simulink model "BswSigProc"  (R2022b)
settings: Solver=FixedStepDiscrete  StartTime=0.0  StopTime=10.0  FixedStep=0.01  RelTol=1e-3
model workspace (MAT data in the model file):
  NvMBlockRecordSignal : Simulink.Signal (value not decoded)
  NvMBlockRecordSignalInit : Simulink.Parameter (value not decoded)
symbols that are objects in the model workspace (value not decoded): NvMBlockRecordSignalInit
(part 2 of 2)

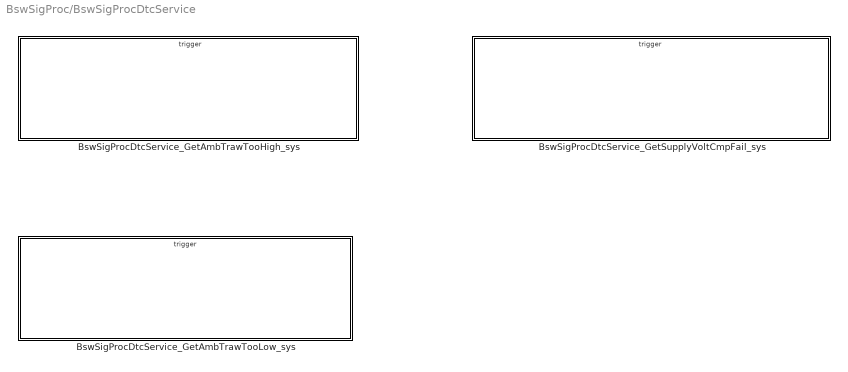
== system BswSigProc/BswSigProcDtcService ==
  BswSigProcDtcService_GetAmbTrawTooHigh_sys : Subsystem
  BswSigProcDtcService_GetAmbTrawTooLow_sys : Subsystem
  BswSigProcDtcService_GetSupplyVoltCmpFail_sys : Subsystem

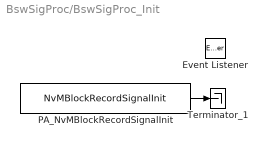
== system BswSigProc/BswSigProc_Init ==
  Event Listener : EventListener  EventName=reset
  PA_NvMBlockRecordSignalInit : Constant  Value=NvMBlockRecordSignalInit
  Terminator_1 : Terminator
  wires:
  PA_NvMBlockRecordSignalInit/1 -> Terminator_1/1

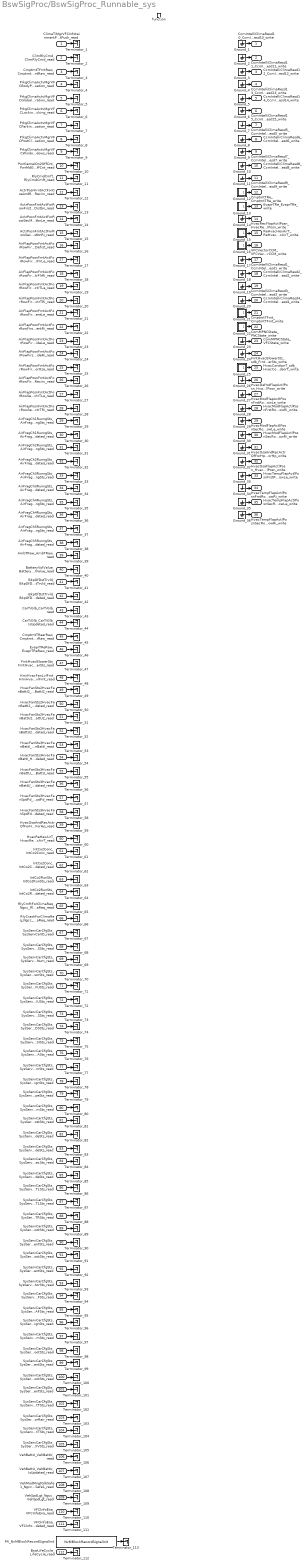
== system BswSigProc/BswSigProc_Runnable_sys ==
  ClimaTiMgrVFCInfotainmentPush_ClimaTiMgrVFCInfotainmentPush_read : Inport
  ClimRlyCmd_ClimRlyCmd_read : Inport  Port=2
  CmptmtTFrntRaw_CmptmtTFrntRaw_read : Inport  Port=3
  PrkgClimaActvMgrVFCBodyPreClimatisation_PrkgClimaActvMgrVFCBodyPreClimatisation_read : Inport  Port=4
  PrkgClimaActvMgrVFCGlobalShortDuration_PrkgClimaActvMgrVFCGlobalShortDuration_read : Inport  Port=5
  PrkgClimaActvMgrVFCLocking_PrkgClimaActvMgrVFCLocking_read : Inport  Port=6
  PrkgClimaActvMgrVFCParkingDrivingClimatization_PrkgClimaActvMgrVFCParkingDrivingClimatization_read : Inport  Port=7
  PrkgClimaActvMgrVFCPostClimatisation_PrkgClimaActvMgrVFCPostClimatisation_read : Inport  Port=8
  PrkgClimaActvMgrVFCWindows_PrkgClimaActvMgrVFCWindows_read : Inport  Port=9
  PwrGernalOn2OffCnt_PwrMdGernalOn2OffCnt_read : Inport  Port=10
  RlyCmdCmft_RlyCmdCmft_read : Inport  Port=11
  ActrPosnFrntActForOsaAndRecirc_ActrPosnFrntActForOsaAndRecirc_read : Inport  Port=12
  ActrPosnFrntActForRowFirstModDistbn_ActrPosnFrntActForRowFirstModDistbn_read : Inport  Port=13
  ActrPosnFrntActForRowSecModDistbnLe_ActrPosnFrntActForRowSecModDistbnLe_read : Inport  Port=14
  ActrPosnFrntActForRowSecModDistbnRi_ActrPosnFrntActForRowSecModDistbnRi_read : Inport  Port=15
  AirFlapPosnFrntActForRowFirstForActrDefrst_AirFlapPosnFrntActForRowFirstForActrDefrst_read : Inport  Port=16
  AirFlapPosnFrntActForRowFirstForActrFlrLe_AirFlapPosnFrntActForRowFirstForActrFlrLe_read : Inport  Port=17
  AirFlapPosnFrntActForRowFirstForActrFlrRi_AirFlapPosnFrntActForRowFirstForActrFlrRi_read : Inport  Port=18
  AirFlapPosnFrntActForRowFirstForActrTLe_AirFlapPosnFrntActForRowFirstForActrTLe_read : Inport  Port=19
  AirFlapPosnFrntActForRowFirstForActrTRi_AirFlapPosnFrntActForRowFirstForActrTRi_read : Inport  Port=20
  AirFlapPosnFrntActForRowFirstForActrVentLe_AirFlapPosnFrntActForRowFirstForActrVentLe_read : Inport  Port=21
  AirFlapPosnFrntActForRowFirstForActrVentRi_AirFlapPosnFrntActForRowFirstForActrVentRi_read : Inport  Port=22
  AirFlapPosnFrntActForRowFirstForActrVentSideLe_AirFlapPosnFrntActForRowFirstForActrVentSideLe_read : Inport  Port=23
  AirFlapPosnFrntActForRowFirstForActrVentSideRi_AirFlapPosnFrntActForRowFirstForActrVentSideRi_read : Inport  Port=24
  AirFlapPosnFrntActForRowFirstForOsa_AirFlapPosnFrntActForRowFirstForOsa_read : Inport  Port=25
  AirFlapPosnFrntActForRowFirstForRecirc_AirFlapPosnFrntActForRowFirstForRecirc_read : Inport  Port=26
  AirFlapPosnFrntActForRowSecForActrTLe_AirFlapPosnFrntActForRowSecForActrTLe_read : Inport  Port=27
  AirFlapPosnFrntActForRowSecForActrTRi_AirFlapPosnFrntActForRowSecForActrTRi_read : Inport  Port=28
  AirFragCh1RunngSts_AirFragCh1RunngSts_read : Inport  Port=29
  AirFragCh1RunngSts_AirFragCh1RunngSts_IsUpdated_read : Inport  Port=30
  AirFragCh2RunngSts_AirFragCh2RunngSts_read : Inport  Port=31
  AirFragCh2RunngSts_AirFragCh2RunngSts_IsUpdated_read : Inport  Port=32
  AirFragCh3RunngSts_AirFragCh3RunngSts_read : Inport  Port=33
  AirFragCh3RunngSts_AirFragCh3RunngSts_IsUpdated_read : Inport  Port=34
  AirFragCh4RunngSts_AirFragCh4RunngSts_read : Inport  Port=35
  AirFragCh4RunngSts_AirFragCh4RunngSts_IsUpdated_read : Inport  Port=36
  AirFragCh5RunngSts_AirFragCh5RunngSts_read : Inport  Port=37
  AirFragCh5RunngSts_AirFragCh5RunngSts_IsUpdated_read : Inport  Port=38
  AmbTRaw_AmbTRaw_read : Inport  Port=39
  BatteryVolValue_BatteryVolValue_read : Inport  Port=40
  BkpOfDstTrvld_BkpOfDstTrvld_read : Inport  Port=41
  BkpOfDstTrvld_BkpOfDstTrvld_IsUpdated_read : Inport  Port=42
  CarTiGlb_CarTiGlb_read : Inport  Port=43
  CarTiGlb_CarTiGlb_IsUpdated_read : Inport  Port=44
  CmptmtTRearRaw_CmptmtTRearRaw_read : Inport  Port=45
  EvaprTReRaw_EvaprTReRaw_read : Inport  Port=46
  FrntHvacBlowerSts_FrntHvacBlowerSts_read : Inport  Port=47
  HmiHvacFanLvlFrnt_HmiHvacFanLvlFrnt_read : Inport  Port=48
  HvacFanSts2HvacFanBattI2_HvacFanSts2HvacFanBattI2_read : Inport  Port=49
  HvacFanSts2HvacFanBattI2_HvacFanSts2HvacFanBattI2_IsUpdated_read : Inport  Port=50
  HvacFanSts2HvacFanBattU2_HvacFanSts2HvacFanBattU2_read : Inport  Port=51
  HvacFanSts2HvacFanBattU2_HvacFanSts2HvacFanBattU2_IsUpdated_read : Inport  Port=52
  HvacFanSts3HvacFanBattI_HvacFanSts3HvacFanBattI_read : Inport  Port=53
  HvacFanSts3HvacFanBattI_HvacFanSts3HvacFanBattI_IsUpdated_read : Inport  Port=54
  HvacFanSts3HvacFanBattU_HvacFanSts3HvacFanBattU_read : Inport  Port=55
  HvacFanSts3HvacFanBattU_HvacFanSts3HvacFanBattU_IsUpdated_read : Inport  Port=56
  HvacFanSts3HvacFanSpdFd_HvacFanSts3HvacFanSpdFd_read : Inport  Port=57
  HvacFanSts3HvacFanSpdFd_HvacFanSts3HvacFanSpdFd_IsUpdated_read : Inport  Port=58
  HvacOsaAndRecActrQfForHp_HvacOsaAndRecActrQfForHp_read : Inport  Port=59
  HvacReHexAirT_HvacReHexAirT_read : Inport  Port=60
  IntCo2Conc_IntCo2Conc_read : Inport  Port=61
  IntCo2Conc_IntCo2Conc_IsUpdated_read : Inport  Port=62
  IntCo2RunSts_IntCo2RunSts_read : Inport  Port=63
  IntCo2RunSts_IntCo2RunSts_IsUpdated_read : Inport  Port=64
  RlyCmftForClimaReq_Ngcc_RlyCmftForClimaReq_read : Inport  Port=65
  RlyCrashForClimaReq_Ngcc_RlyCrashForClimaReq_read : Inport  Port=66
  SysServCarCfgSts_SysServCarID_read : Inport  Port=67
  SysServCarCfgSts_SysServCCPAQSSts_read : Inport  Port=68
  SysServCarCfgSts_SysServCCPBGMNum_read : Inport  Port=69
  SysServCarCfgSts_SysServCCPHEXSensorSts_read : Inport  Port=70
  SysServCarCfgSts_SysServCCPLin2AFUSts_read : Inport  Port=71
  SysServCarCfgSts_SysServCCPLin2AIUSts_read : Inport  Port=72
  SysServCarCfgSts_SysServCCPLin2AQSSts_read : Inport  Port=73
  SysServCarCfgSts_SysServCCPLin2CDSSts_read : Inport  Port=74
  SysServCarCfgSts_SysServCCPLin2PMSISts_read : Inport  Port=75
  SysServCarCfgSts_SysServCCPLin3VGASts_read : Inport  Port=76
  SysServCarCfgSts_SysServDUAirVentSnrSts_read : Inport  Port=77
  SysServCarCfgSts_SysServDUBlowerPwmSignSts_read : Inport  Port=78
  SysServCarCfgSts_SysServDUBlowerPwmSignTypeSts_read : Inport  Port=79
  SysServCarCfgSts_SysServDUBlowerPwmSts_read : Inport  Port=80
  SysServCarCfgSts_SysServDUDCDefrostSts_read : Inport  Port=81
  SysServCarCfgSts_SysServDUDCFLMdeSts_read : Inport  Port=82
  SysServCarCfgSts_SysServDUDCModeSts_read : Inport  Port=83
  SysServCarCfgSts_SysServDUDCRecSts_read : Inport  Port=84
  SysServCarCfgSts_SysServDUDCSModeSts_read : Inport  Port=85
  SysServCarCfgSts_SysServDUDCSTLSts_read : Inport  Port=86
  SysServCarCfgSts_SysServDUDCTLSts_read : Inport  Port=87
  SysServCarCfgSts_SysServDUDCTRSts_read : Inport  Port=88
  SysServCarCfgSts_SysServDUFLFootSts_read : Inport  Port=89
  SysServCarCfgSts_SysServDUFLVentSts_read : Inport  Port=90
  SysServCarCfgSts_SysServDUFRFootSts_read : Inport  Port=91
  SysServCarCfgSts_SysServDUFRVentSts_read : Inport  Port=92
  SysServCarCfgSts_SysServDUIncarMotorSts_read : Inport  Port=93
  SysServCarCfgSts_SysServDULin1HBMFSts_read : Inport  Port=94
  SysServCarCfgSts_SysServDULin4HFAFSts_read : Inport  Port=95
  SysServCarCfgSts_SysServDUReBlowerPwmSignSts_read : Inport  Port=96
  SysServCarCfgSts_SysServDUReBlowerPwmSts_read : Inport  Port=97
  SysServCarCfgSts_SysServDUSFLFootSts_read : Inport  Port=98
  SysServCarCfgSts_SysServDUSFLVentSts_read : Inport  Port=99
  SysServCarCfgSts_SysServDUSFRFootSts_read : Inport  Port=100
  SysServCarCfgSts_SysServDUSFRVentSts_read : Inport  Port=101
  SysServCarCfgSts_SysServDUSnsrForFCmptmtTSts_read : Inport  Port=102
  SysServCarCfgSts_SysServDUSnsrForRair_read : Inport  Port=103
  SysServCarCfgSts_SysServDUSnsrForRCmptmtTSts_read : Inport  Port=104
  SysServCarCfgSts_SysServLowSideRETXVSts_read : Inport  Port=105
  VehBattU_VehBattU_read : Inport  Port=106
  VehBattU_VehBattU_IsUpdated_read : Inport  Port=107
  VehModMngtGlbSafe1_Ngcc_VehModMngtGlbSafe1_read : Inport  Port=108
  VehSpdLgt_Ngcc_VehSpdLgt_read : Inport  Port=109
  VFCInfoEna_VFCInfoEna_read : Inport  Port=110
  VFCInfoEna_VFCInfoEna_IsUpdated_read : Inport  Port=111
  BswLifeCycle_LifeCycle_read : Inport  Port=112
  function : TriggerPort  TriggerType=function-call  VariantControl=(inherit)  SampleTimeType=periodic  SampleTime=-1
  Ground_{1..11,14,17..20,23..24,26..36} (29 alike) : Ground
  Ground_12 : Subsystem
  Ground_13 : Subsystem
  Ground_15 : Subsystem
  Ground_16 : Subsystem
  Ground_21 : Subsystem
  Ground_22 : Subsystem
  Ground_25 : Subsystem
  PA_NvMBlockRecordSignalInit : Constant  Value=NvMBlockRecordSignalInit
  Terminator_{1..113} (113 alike) : Terminator
  CcmIntelliClimaResd10_CcmIntelliClimaResd10_write : Outport
      settings: EnsureOutportIsVirtual=on
  CcmIntelliClimaResd11_CcmIntelliClimaResd11_write : Outport  Port=2
      settings: EnsureOutportIsVirtual=on
  CcmIntelliClimaResd12_CcmIntelliClimaResd12_write : Outport  Port=3
      settings: EnsureOutportIsVirtual=on
  CcmIntelliClimaResd13_CcmIntelliClimaResd13_write : Outport  Port=4
      settings: EnsureOutportIsVirtual=on
  CcmIntelliClimaResd14_CcmIntelliClimaResd14_write : Outport  Port=5
      settings: EnsureOutportIsVirtual=on
  CcmIntelliClimaResd15_CcmIntelliClimaResd15_write : Outport  Port=6
      settings: EnsureOutportIsVirtual=on
  CcmIntelliClimaResd5_CcmIntelliClimaResd5_write : Outport  Port=7
      settings: EnsureOutportIsVirtual=on
  CcmIntelliClimaResd6_CcmIntelliClimaResd6_write : Outport  Port=8
      settings: EnsureOutportIsVirtual=on
  CcmIntelliClimaResd7_CcmIntelliClimaResd7_write : Outport  Port=9
      settings: EnsureOutportIsVirtual=on
  CcmIntelliClimaResd8_CcmIntelliClimaResd8_write : Outport  Port=10
      settings: EnsureOutportIsVirtual=on
  CcmIntelliClimaResd9_CcmIntelliClimaResd9_write : Outport  Port=11
      settings: EnsureOutportIsVirtual=on
  CmptmtTRe_CmptmtTRe_write : Outport  Port=12
      settings: EnsureOutportIsVirtual=on
  EvaprTRe_EvaprTRe_write : Outport  Port=13
      settings: EnsureOutportIsVirtual=on
  HvacRecFlapActlPosn_HvacRecFlapActlPosn_write : Outport  Port=14
      settings: EnsureOutportIsVirtual=on
  ReHvacHexAirT_ReHvacHexAirT_write : Outport  Port=15
      settings: EnsureOutportIsVirtual=on
  VFCVectorCCM_VFCVectorCCM_write : Outport  Port=16
      settings: EnsureOutportIsVirtual=on
  CcmIntelliClimaResd1_CcmIntelliClimaResd1_write : Outport  Port=17
      settings: EnsureOutportIsVirtual=on
  CcmIntelliClimaResd2_CcmIntelliClimaResd2_write : Outport  Port=18
      settings: EnsureOutportIsVirtual=on
  CcmIntelliClimaResd3_CcmIntelliClimaResd3_write : Outport  Port=19
      settings: EnsureOutportIsVirtual=on
  CcmIntelliClimaResd4_CcmIntelliClimaResd4_write : Outport  Port=20
      settings: EnsureOutportIsVirtual=on
  CmptmtTFrnt_CmptmtTFrnt_write : Outport  Port=21
      settings: EnsureOutportIsVirtual=on
  ComMPNCState_PNCState_write : Outport  Port=22
      settings: EnsureOutportIsVirtual=on
  ComMPNCState_VFCState_write : Outport  Port=23
      settings: EnsureOutportIsVirtual=on
  FrntHvacBlowerSts_sdb_FrntHvacBlowerSts_write : Outport  Port=24
      settings: EnsureOutportIsVirtual=on
  HvacCondsorT_sdb_HvacCondsorT_write : Outport  Port=25
      settings: EnsureOutportIsVirtual=on
  HvacDefrstFlapActlPosn_HvacDefrstFlapActlPosn_write : Outport  Port=26
      settings: EnsureOutportIsVirtual=on
  HvacModFlapActlPosnFrstRowLe_HvacModFlapActlPosnFrstRowLe_write : Outport  Port=27
      settings: EnsureOutportIsVirtual=on
  HvacModFlapActlPosnFrstRowRi_HvacModFlapActlPosnFrstRowRi_write : Outport  Port=28
      settings: EnsureOutportIsVirtual=on
  HvacModFlapActlPosnSecRowLe_HvacModFlapActlPosnSecRowLe_write : Outport  Port=29
      settings: EnsureOutportIsVirtual=on
  HvacModFlapActlPosnSecRowRi_HvacModFlapActlPosnSecRowRi_write : Outport  Port=30
      settings: EnsureOutportIsVirtual=on
  HvacOsaAndRecActrQfForHp_sdb_HvacOsaAndRecActrQfForHp_write : Outport  Port=31
      settings: EnsureOutportIsVirtual=on
  HvacOsaFlapActlPosn_HvacOsaFlapActlPosn_write : Outport  Port=32
      settings: EnsureOutportIsVirtual=on
  HvacTempFlapActlPosnFrstRowLe_HvacTempFlapActlPosnFrstRowLe_write : Outport  Port=33
      settings: EnsureOutportIsVirtual=on
  HvacTempFlapActlPosnFrstRowRi_HvacTempFlapActlPosnFrstRowRi_write : Outport  Port=34
      settings: EnsureOutportIsVirtual=on
  HvacTempFlapActlPosnSecRowLe_HvacTempFlapActlPosnSecRowLe_write : Outport  Port=35
      settings: EnsureOutportIsVirtual=on
  HvacTempFlapActlPosnSecRowRi_HvacTempFlapActlPosnSecRowRi_write : Outport  Port=36
      settings: EnsureOutportIsVirtual=on
  wires:
  ClimaTiMgrVFCInfotainmentPush_ClimaTiMgrVFCInfotainmentPush_read/1 -> Terminator_1/1
  ClimRlyCmd_ClimRlyCmd_read/1 -> Terminator_2/1
  CmptmtTFrntRaw_CmptmtTFrntRaw_read/1 -> Terminator_3/1
  PrkgClimaActvMgrVFCBodyPreClimatisation_PrkgClimaActvMgrVFCBodyPreClimatisation_read/1 -> Terminator_4/1
  PrkgClimaActvMgrVFCGlobalShortDuration_PrkgClimaActvMgrVFCGlobalShortDuration_read/1 -> Terminator_5/1
  PrkgClimaActvMgrVFCLocking_PrkgClimaActvMgrVFCLocking_read/1 -> Terminator_6/1
  PrkgClimaActvMgrVFCParkingDrivingClimatization_PrkgClimaActvMgrVFCParkingDrivingClimatization_read/1 -> Terminator_7/1
  PrkgClimaActvMgrVFCPostClimatisation_PrkgClimaActvMgrVFCPostClimatisation_read/1 -> Terminator_8/1
  PrkgClimaActvMgrVFCWindows_PrkgClimaActvMgrVFCWindows_read/1 -> Terminator_9/1
  PwrGernalOn2OffCnt_PwrMdGernalOn2OffCnt_read/1 -> Terminator_10/1
  RlyCmdCmft_RlyCmdCmft_read/1 -> Terminator_11/1
  ActrPosnFrntActForOsaAndRecirc_ActrPosnFrntActForOsaAndRecirc_read/1 -> Terminator_12/1
  ActrPosnFrntActForRowFirstModDistbn_ActrPosnFrntActForRowFirstModDistbn_read/1 -> Terminator_13/1
  ActrPosnFrntActForRowSecModDistbnLe_ActrPosnFrntActForRowSecModDistbnLe_read/1 -> Terminator_14/1
  ActrPosnFrntActForRowSecModDistbnRi_ActrPosnFrntActForRowSecModDistbnRi_read/1 -> Terminator_15/1
  AirFlapPosnFrntActForRowFirstForActrDefrst_AirFlapPosnFrntActForRowFirstForActrDefrst_read/1 -> Terminator_16/1
  AirFlapPosnFrntActForRowFirstForActrFlrLe_AirFlapPosnFrntActForRowFirstForActrFlrLe_read/1 -> Terminator_17/1
  AirFlapPosnFrntActForRowFirstForActrFlrRi_AirFlapPosnFrntActForRowFirstForActrFlrRi_read/1 -> Terminator_18/1
  AirFlapPosnFrntActForRowFirstForActrTLe_AirFlapPosnFrntActForRowFirstForActrTLe_read/1 -> Terminator_19/1
  AirFlapPosnFrntActForRowFirstForActrTRi_AirFlapPosnFrntActForRowFirstForActrTRi_read/1 -> Terminator_20/1
  AirFlapPosnFrntActForRowFirstForActrVentLe_AirFlapPosnFrntActForRowFirstForActrVentLe_read/1 -> Terminator_21/1
  AirFlapPosnFrntActForRowFirstForActrVentRi_AirFlapPosnFrntActForRowFirstForActrVentRi_read/1 -> Terminator_22/1
  AirFlapPosnFrntActForRowFirstForActrVentSideLe_AirFlapPosnFrntActForRowFirstForActrVentSideLe_read/1 -> Terminator_23/1
  AirFlapPosnFrntActForRowFirstForActrVentSideRi_AirFlapPosnFrntActForRowFirstForActrVentSideRi_read/1 -> Terminator_24/1
  AirFlapPosnFrntActForRowFirstForOsa_AirFlapPosnFrntActForRowFirstForOsa_read/1 -> Terminator_25/1
  AirFlapPosnFrntActForRowFirstForRecirc_AirFlapPosnFrntActForRowFirstForRecirc_read/1 -> Terminator_26/1
  AirFlapPosnFrntActForRowSecForActrTLe_AirFlapPosnFrntActForRowSecForActrTLe_read/1 -> Terminator_27/1
  AirFlapPosnFrntActForRowSecForActrTRi_AirFlapPosnFrntActForRowSecForActrTRi_read/1 -> Terminator_28/1
  AirFragCh1RunngSts_AirFragCh1RunngSts_read/1 -> Terminator_29/1
  AirFragCh1RunngSts_AirFragCh1RunngSts_IsUpdated_read/1 -> Terminator_30/1
  AirFragCh2RunngSts_AirFragCh2RunngSts_read/1 -> Terminator_31/1
  AirFragCh2RunngSts_AirFragCh2RunngSts_IsUpdated_read/1 -> Terminator_32/1
  AirFragCh3RunngSts_AirFragCh3RunngSts_read/1 -> Terminator_33/1
  AirFragCh3RunngSts_AirFragCh3RunngSts_IsUpdated_read/1 -> Terminator_34/1
  AirFragCh4RunngSts_AirFragCh4RunngSts_read/1 -> Terminator_35/1
  AirFragCh4RunngSts_AirFragCh4RunngSts_IsUpdated_read/1 -> Terminator_36/1
  AirFragCh5RunngSts_AirFragCh5RunngSts_read/1 -> Terminator_37/1
  AirFragCh5RunngSts_AirFragCh5RunngSts_IsUpdated_read/1 -> Terminator_38/1
  AmbTRaw_AmbTRaw_read/1 -> Terminator_39/1
  BatteryVolValue_BatteryVolValue_read/1 -> Terminator_40/1
  BkpOfDstTrvld_BkpOfDstTrvld_read/1 -> Terminator_41/1
  BkpOfDstTrvld_BkpOfDstTrvld_IsUpdated_read/1 -> Terminator_42/1
  CarTiGlb_CarTiGlb_read/1 -> Terminator_43/1
  CarTiGlb_CarTiGlb_IsUpdated_read/1 -> Terminator_44/1
  CmptmtTRearRaw_CmptmtTRearRaw_read/1 -> Terminator_45/1
  EvaprTReRaw_EvaprTReRaw_read/1 -> Terminator_46/1
  FrntHvacBlowerSts_FrntHvacBlowerSts_read/1 -> Terminator_47/1
  HmiHvacFanLvlFrnt_HmiHvacFanLvlFrnt_read/1 -> Terminator_48/1
  HvacFanSts2HvacFanBattI2_HvacFanSts2HvacFanBattI2_read/1 -> Terminator_49/1
  HvacFanSts2HvacFanBattI2_HvacFanSts2HvacFanBattI2_IsUpdated_read/1 -> Terminator_50/1
  HvacFanSts2HvacFanBattU2_HvacFanSts2HvacFanBattU2_read/1 -> Terminator_51/1
  HvacFanSts2HvacFanBattU2_HvacFanSts2HvacFanBattU2_IsUpdated_read/1 -> Terminator_52/1
  HvacFanSts3HvacFanBattI_HvacFanSts3HvacFanBattI_read/1 -> Terminator_53/1
  HvacFanSts3HvacFanBattI_HvacFanSts3HvacFanBattI_IsUpdated_read/1 -> Terminator_54/1
  HvacFanSts3HvacFanBattU_HvacFanSts3HvacFanBattU_read/1 -> Terminator_55/1
  HvacFanSts3HvacFanBattU_HvacFanSts3HvacFanBattU_IsUpdated_read/1 -> Terminator_56/1
  HvacFanSts3HvacFanSpdFd_HvacFanSts3HvacFanSpdFd_read/1 -> Terminator_57/1
  HvacFanSts3HvacFanSpdFd_HvacFanSts3HvacFanSpdFd_IsUpdated_read/1 -> Terminator_58/1
  HvacOsaAndRecActrQfForHp_HvacOsaAndRecActrQfForHp_read/1 -> Terminator_59/1
  HvacReHexAirT_HvacReHexAirT_read/1 -> Terminator_60/1
  IntCo2Conc_IntCo2Conc_read/1 -> Terminator_61/1
  IntCo2Conc_IntCo2Conc_IsUpdated_read/1 -> Terminator_62/1
  IntCo2RunSts_IntCo2RunSts_read/1 -> Terminator_63/1
  IntCo2RunSts_IntCo2RunSts_IsUpdated_read/1 -> Terminator_64/1
  RlyCmftForClimaReq_Ngcc_RlyCmftForClimaReq_read/1 -> Terminator_65/1
  RlyCrashForClimaReq_Ngcc_RlyCrashForClimaReq_read/1 -> Terminator_66/1
  SysServCarCfgSts_SysServCarID_read/1 -> Terminator_67/1
  SysServCarCfgSts_SysServCCPAQSSts_read/1 -> Terminator_68/1
  SysServCarCfgSts_SysServCCPBGMNum_read/1 -> Terminator_69/1
  SysServCarCfgSts_SysServCCPHEXSensorSts_read/1 -> Terminator_70/1
  SysServCarCfgSts_SysServCCPLin2AFUSts_read/1 -> Terminator_71/1
  SysServCarCfgSts_SysServCCPLin2AIUSts_read/1 -> Terminator_72/1
  SysServCarCfgSts_SysServCCPLin2AQSSts_read/1 -> Terminator_73/1
  SysServCarCfgSts_SysServCCPLin2CDSSts_read/1 -> Terminator_74/1
  SysServCarCfgSts_SysServCCPLin2PMSISts_read/1 -> Terminator_75/1
  SysServCarCfgSts_SysServCCPLin3VGASts_read/1 -> Terminator_76/1
  SysServCarCfgSts_SysServDUAirVentSnrSts_read/1 -> Terminator_77/1
  SysServCarCfgSts_SysServDUBlowerPwmSignSts_read/1 -> Terminator_78/1
  SysServCarCfgSts_SysServDUBlowerPwmSignTypeSts_read/1 -> Terminator_79/1
  SysServCarCfgSts_SysServDUBlowerPwmSts_read/1 -> Terminator_80/1
  SysServCarCfgSts_SysServDUDCDefrostSts_read/1 -> Terminator_81/1
  SysServCarCfgSts_SysServDUDCFLMdeSts_read/1 -> Terminator_82/1
  SysServCarCfgSts_SysServDUDCModeSts_read/1 -> Terminator_83/1
  SysServCarCfgSts_SysServDUDCRecSts_read/1 -> Terminator_84/1
  SysServCarCfgSts_SysServDUDCSModeSts_read/1 -> Terminator_85/1
  SysServCarCfgSts_SysServDUDCSTLSts_read/1 -> Terminator_86/1
  SysServCarCfgSts_SysServDUDCTLSts_read/1 -> Terminator_87/1
  SysServCarCfgSts_SysServDUDCTRSts_read/1 -> Terminator_88/1
  SysServCarCfgSts_SysServDUFLFootSts_read/1 -> Terminator_89/1
  SysServCarCfgSts_SysServDUFLVentSts_read/1 -> Terminator_90/1
  SysServCarCfgSts_SysServDUFRFootSts_read/1 -> Terminator_91/1
  SysServCarCfgSts_SysServDUFRVentSts_read/1 -> Terminator_92/1
  SysServCarCfgSts_SysServDUIncarMotorSts_read/1 -> Terminator_93/1
  SysServCarCfgSts_SysServDULin1HBMFSts_read/1 -> Terminator_94/1
  SysServCarCfgSts_SysServDULin4HFAFSts_read/1 -> Terminator_95/1
  SysServCarCfgSts_SysServDUReBlowerPwmSignSts_read/1 -> Terminator_96/1
  SysServCarCfgSts_SysServDUReBlowerPwmSts_read/1 -> Terminator_97/1
  SysServCarCfgSts_SysServDUSFLFootSts_read/1 -> Terminator_98/1
  SysServCarCfgSts_SysServDUSFLVentSts_read/1 -> Terminator_99/1
  SysServCarCfgSts_SysServDUSFRFootSts_read/1 -> Terminator_100/1
  SysServCarCfgSts_SysServDUSFRVentSts_read/1 -> Terminator_101/1
  SysServCarCfgSts_SysServDUSnsrForFCmptmtTSts_read/1 -> Terminator_102/1
  SysServCarCfgSts_SysServDUSnsrForRair_read/1 -> Terminator_103/1
  SysServCarCfgSts_SysServDUSnsrForRCmptmtTSts_read/1 -> Terminator_104/1
  SysServCarCfgSts_SysServLowSideRETXVSts_read/1 -> Terminator_105/1
  VehBattU_VehBattU_read/1 -> Terminator_106/1
  VehBattU_VehBattU_IsUpdated_read/1 -> Terminator_107/1
  VehModMngtGlbSafe1_Ngcc_VehModMngtGlbSafe1_read/1 -> Terminator_108/1
  VehSpdLgt_Ngcc_VehSpdLgt_read/1 -> Terminator_109/1
  VFCInfoEna_VFCInfoEna_read/1 -> Terminator_110/1
  VFCInfoEna_VFCInfoEna_IsUpdated_read/1 -> Terminator_111/1
  BswLifeCycle_LifeCycle_read/1 -> Terminator_112/1
  PA_NvMBlockRecordSignalInit/1 -> Terminator_113/1
  Ground_1/1 -> CcmIntelliClimaResd10_CcmIntelliClimaResd10_write/1
  Ground_2/1 -> CcmIntelliClimaResd11_CcmIntelliClimaResd11_write/1
  Ground_3/1 -> CcmIntelliClimaResd12_CcmIntelliClimaResd12_write/1
  Ground_4/1 -> CcmIntelliClimaResd13_CcmIntelliClimaResd13_write/1
  Ground_5/1 -> CcmIntelliClimaResd14_CcmIntelliClimaResd14_write/1
  Ground_6/1 -> CcmIntelliClimaResd15_CcmIntelliClimaResd15_write/1
  Ground_7/1 -> CcmIntelliClimaResd5_CcmIntelliClimaResd5_write/1
  Ground_8/1 -> CcmIntelliClimaResd6_CcmIntelliClimaResd6_write/1
  Ground_9/1 -> CcmIntelliClimaResd7_CcmIntelliClimaResd7_write/1
  Ground_10/1 -> CcmIntelliClimaResd8_CcmIntelliClimaResd8_write/1
  Ground_11/1 -> CcmIntelliClimaResd9_CcmIntelliClimaResd9_write/1
  Ground_12/1(Out1) -> CmptmtTRe_CmptmtTRe_write/1
  Ground_13/1(Out1) -> EvaprTRe_EvaprTRe_write/1
  Ground_14/1 -> HvacRecFlapActlPosn_HvacRecFlapActlPosn_write/1
  Ground_15/1(Out1) -> ReHvacHexAirT_ReHvacHexAirT_write/1
  Ground_16/1(Out1) -> VFCVectorCCM_VFCVectorCCM_write/1
  Ground_17/1 -> CcmIntelliClimaResd1_CcmIntelliClimaResd1_write/1
  Ground_18/1 -> CcmIntelliClimaResd2_CcmIntelliClimaResd2_write/1
  Ground_19/1 -> CcmIntelliClimaResd3_CcmIntelliClimaResd3_write/1
  Ground_20/1 -> CcmIntelliClimaResd4_CcmIntelliClimaResd4_write/1
  Ground_21/1(Out1) -> CmptmtTFrnt_CmptmtTFrnt_write/1
  Ground_22/1(Out1) -> ComMPNCState_PNCState_write/1
  Ground_23/1 -> ComMPNCState_VFCState_write/1
  Ground_24/1 -> FrntHvacBlowerSts_sdb_FrntHvacBlowerSts_write/1
  Ground_25/1(Out1) -> HvacCondsorT_sdb_HvacCondsorT_write/1
  Ground_26/1 -> HvacDefrstFlapActlPosn_HvacDefrstFlapActlPosn_write/1
  Ground_27/1 -> HvacModFlapActlPosnFrstRowLe_HvacModFlapActlPosnFrstRowLe_write/1
  Ground_28/1 -> HvacModFlapActlPosnFrstRowRi_HvacModFlapActlPosnFrstRowRi_write/1
  Ground_29/1 -> HvacModFlapActlPosnSecRowLe_HvacModFlapActlPosnSecRowLe_write/1
  Ground_30/1 -> HvacModFlapActlPosnSecRowRi_HvacModFlapActlPosnSecRowRi_write/1
  Ground_31/1 -> HvacOsaAndRecActrQfForHp_sdb_HvacOsaAndRecActrQfForHp_write/1
  Ground_32/1 -> HvacOsaFlapActlPosn_HvacOsaFlapActlPosn_write/1
  Ground_33/1 -> HvacTempFlapActlPosnFrstRowLe_HvacTempFlapActlPosnFrstRowLe_write/1
  Ground_34/1 -> HvacTempFlapActlPosnFrstRowRi_HvacTempFlapActlPosnFrstRowRi_write/1
  Ground_35/1 -> HvacTempFlapActlPosnSecRowLe_HvacTempFlapActlPosnSecRowLe_write/1
  Ground_36/1 -> HvacTempFlapActlPosnSecRowRi_HvacTempFlapActlPosnSecRowRi_write/1

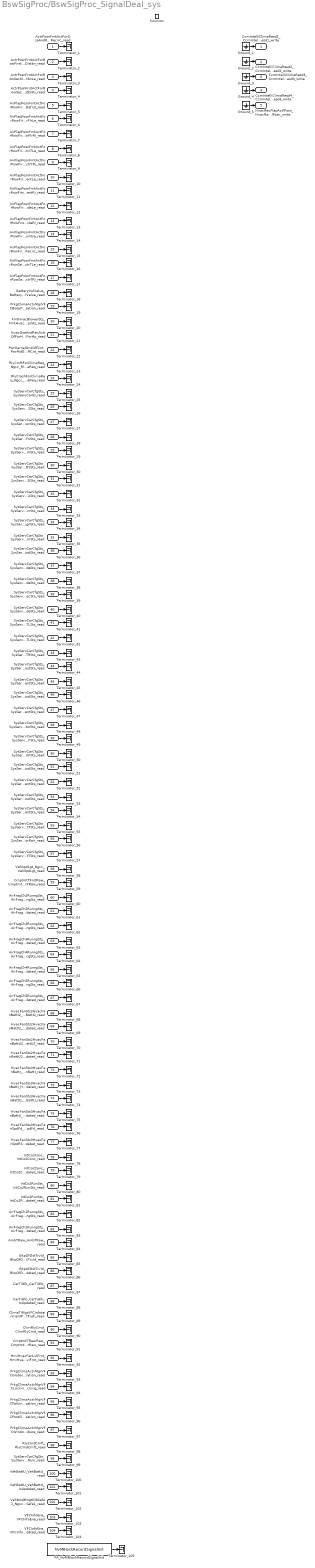
== system BswSigProc/BswSigProc_SignalDeal_sys ==
  ActrPosnFrntActForOsaAndRecirc_ActrPosnFrntActForOsaAndRecirc_read : Inport
  ActrPosnFrntActForRowFirstModDistbn_ActrPosnFrntActForRowFirstModDistbn_read : Inport  Port=2
  ActrPosnFrntActForRowSecModDistbnLe_ActrPosnFrntActForRowSecModDistbnLe_read : Inport  Port=3
  ActrPosnFrntActForRowSecModDistbnRi_ActrPosnFrntActForRowSecModDistbnRi_read : Inport  Port=4
  AirFlapPosnFrntActForRowFirstForActrDefrst_AirFlapPosnFrntActForRowFirstForActrDefrst_read : Inport  Port=5
  AirFlapPosnFrntActForRowFirstForActrFlrLe_AirFlapPosnFrntActForRowFirstForActrFlrLe_read : Inport  Port=6
  AirFlapPosnFrntActForRowFirstForActrFlrRi_AirFlapPosnFrntActForRowFirstForActrFlrRi_read : Inport  Port=7
  AirFlapPosnFrntActForRowFirstForActrTLe_AirFlapPosnFrntActForRowFirstForActrTLe_read : Inport  Port=8
  AirFlapPosnFrntActForRowFirstForActrTRi_AirFlapPosnFrntActForRowFirstForActrTRi_read : Inport  Port=9
  AirFlapPosnFrntActForRowFirstForActrVentLe_AirFlapPosnFrntActForRowFirstForActrVentLe_read : Inport  Port=10
  AirFlapPosnFrntActForRowFirstForActrVentRi_AirFlapPosnFrntActForRowFirstForActrVentRi_read : Inport  Port=11
  AirFlapPosnFrntActForRowFirstForActrVentSideLe_AirFlapPosnFrntActForRowFirstForActrVentSideLe_read : Inport  Port=12
  AirFlapPosnFrntActForRowFirstForActrVentSideRi_AirFlapPosnFrntActForRowFirstForActrVentSideRi_read : Inport  Port=13
  AirFlapPosnFrntActForRowFirstForOsa_AirFlapPosnFrntActForRowFirstForOsa_read : Inport  Port=14
  AirFlapPosnFrntActForRowFirstForRecirc_AirFlapPosnFrntActForRowFirstForRecirc_read : Inport  Port=15
  AirFlapPosnFrntActForRowSecForActrTLe_AirFlapPosnFrntActForRowSecForActrTLe_read : Inport  Port=16
  AirFlapPosnFrntActForRowSecForActrTRi_AirFlapPosnFrntActForRowSecForActrTRi_read : Inport  Port=17
  BatteryVolValue_BatteryVolValue_read : Inport  Port=18
  PrkgClimaActvMgrVFCBodyPreClimatisation_PrkgClimaActvMgrVFCBodyPreClimatisation_read : Inport  Port=19
  FrntHvacBlowerSts_FrntHvacBlowerSts_read : Inport  Port=20
  HvacOsaAndRecActrQfForHp_HvacOsaAndRecActrQfForHp_read : Inport  Port=21
  PwrGernalOn2OffCnt_PwrMdGernalOn2OffCnt_read : Inport  Port=22
  RlyCmftForClimaReq_Ngcc_RlyCmftForClimaReq_read : Inport  Port=23
  RlyCrashForClimaReq_Ngcc_RlyCrashForClimaReq_read : Inport  Port=24
  SysServCarCfgSts_SysServCarID_read : Inport  Port=25
  SysServCarCfgSts_SysServCCPAQSSts_read : Inport  Port=26
  SysServCarCfgSts_SysServCCPHEXSensorSts_read : Inport  Port=27
  SysServCarCfgSts_SysServCCPLin2AFUSts_read : Inport  Port=28
  SysServCarCfgSts_SysServCCPLin2AIUSts_read : Inport  Port=29
  SysServCarCfgSts_SysServCCPLin2CDSSts_read : Inport  Port=30
  SysServCarCfgSts_SysServCCPLin2PMSISts_read : Inport  Port=31
  SysServCarCfgSts_SysServCCPLin3VGASts_read : Inport  Port=32
  SysServCarCfgSts_SysServDUAirVentSnrSts_read : Inport  Port=33
  SysServCarCfgSts_SysServDUBlowerPwmSignSts_read : Inport  Port=34
  SysServCarCfgSts_SysServDUBlowerPwmSts_read : Inport  Port=35
  SysServCarCfgSts_SysServDUDCDefrostSts_read : Inport  Port=36
  SysServCarCfgSts_SysServDUDCFLMdeSts_read : Inport  Port=37
  SysServCarCfgSts_SysServDUDCModeSts_read : Inport  Port=38
  SysServCarCfgSts_SysServDUDCRecSts_read : Inport  Port=39
  SysServCarCfgSts_SysServDUDCSModeSts_read : Inport  Port=40
  SysServCarCfgSts_SysServDUDCSTLSts_read : Inport  Port=41
  SysServCarCfgSts_SysServDUDCTLSts_read : Inport  Port=42
  SysServCarCfgSts_SysServDUDCTRSts_read : Inport  Port=43
  SysServCarCfgSts_SysServDUFLFootSts_read : Inport  Port=44
  SysServCarCfgSts_SysServDUFLVentSts_read : Inport  Port=45
  SysServCarCfgSts_SysServDUFRFootSts_read : Inport  Port=46
  SysServCarCfgSts_SysServDUFRVentSts_read : Inport  Port=47
  SysServCarCfgSts_SysServDUIncarMotorSts_read : Inport  Port=48
  SysServCarCfgSts_SysServDULin1HBMFSts_read : Inport  Port=49
  SysServCarCfgSts_SysServDULin4HFAFSts_read : Inport  Port=50
  SysServCarCfgSts_SysServDUSFLFootSts_read : Inport  Port=51
  SysServCarCfgSts_SysServDUSFLVentSts_read : Inport  Port=52
  SysServCarCfgSts_SysServDUSFRFootSts_read : Inport  Port=53
  SysServCarCfgSts_SysServDUSFRVentSts_read : Inport  Port=54
  SysServCarCfgSts_SysServDUSnsrForFCmptmtTSts_read : Inport  Port=55
  SysServCarCfgSts_SysServDUSnsrForRair_read : Inport  Port=56
  SysServCarCfgSts_SysServDUSnsrForRCmptmtTSts_read : Inport  Port=57
  VehSpdLgt_Ngcc_VehSpdLgt_read : Inport  Port=58
  CmptmtTFrntRaw_CmptmtTFrntRaw_read : Inport  Port=59
  AirFragCh2RunngSts_AirFragCh2RunngSts_read : Inport  Port=60
  AirFragCh2RunngSts_AirFragCh2RunngSts_IsUpdated_read : Inport  Port=61
  AirFragCh3RunngSts_AirFragCh3RunngSts_read : Inport  Port=62
  AirFragCh3RunngSts_AirFragCh3RunngSts_IsUpdated_read : Inport  Port=63
  AirFragCh4RunngSts_AirFragCh4RunngSts_read : Inport  Port=64
  AirFragCh4RunngSts_AirFragCh4RunngSts_IsUpdated_read : Inport  Port=65
  AirFragCh5RunngSts_AirFragCh5RunngSts_read : Inport  Port=66
  AirFragCh5RunngSts_AirFragCh5RunngSts_IsUpdated_read : Inport  Port=67
  HvacFanSts2HvacFanBattI2_HvacFanSts2HvacFanBattI2_read : Inport  Port=68
  HvacFanSts2HvacFanBattI2_HvacFanSts2HvacFanBattI2_IsUpdated_read : Inport  Port=69
  HvacFanSts2HvacFanBattU2_HvacFanSts2HvacFanBattU2_read : Inport  Port=70
  HvacFanSts2HvacFanBattU2_HvacFanSts2HvacFanBattU2_IsUpdated_read : Inport  Port=71
  HvacFanSts3HvacFanBattI_HvacFanSts3HvacFanBattI_read : Inport  Port=72
  HvacFanSts3HvacFanBattI_HvacFanSts3HvacFanBattI_IsUpdated_read : Inport  Port=73
  HvacFanSts3HvacFanBattU_HvacFanSts3HvacFanBattU_read : Inport  Port=74
  HvacFanSts3HvacFanBattU_HvacFanSts3HvacFanBattU_IsUpdated_read : Inport  Port=75
  HvacFanSts3HvacFanSpdFd_HvacFanSts3HvacFanSpdFd_read : Inport  Port=76
  HvacFanSts3HvacFanSpdFd_HvacFanSts3HvacFanSpdFd_IsUpdated_read : Inport  Port=77
  IntCo2Conc_IntCo2Conc_read : Inport  Port=78
  IntCo2Conc_IntCo2Conc_IsUpdated_read : Inport  Port=79
  IntCo2RunSts_IntCo2RunSts_read : Inport  Port=80
  IntCo2RunSts_IntCo2RunSts_IsUpdated_read : Inport  Port=81
  AirFragCh1RunngSts_AirFragCh1RunngSts_read : Inport  Port=82
  AirFragCh1RunngSts_AirFragCh1RunngSts_IsUpdated_read : Inport  Port=83
  AmbTRaw_AmbTRaw_read : Inport  Port=84
  BkpOfDstTrvld_BkpOfDstTrvld_read : Inport  Port=85
  BkpOfDstTrvld_BkpOfDstTrvld_IsUpdated_read : Inport  Port=86
  CarTiGlb_CarTiGlb_read : Inport  Port=87
  CarTiGlb_CarTiGlb_IsUpdated_read : Inport  Port=88
  ClimaTiMgrVFCInfotainmentPush_ClimaTiMgrVFCInfotainmentPush_read : Inport  Port=89
  ClimRlyCmd_ClimRlyCmd_read : Inport  Port=90
  CmptmtTRearRaw_CmptmtTRearRaw_read : Inport  Port=91
  HmiHvacFanLvlFrnt_HmiHvacFanLvlFrnt_read : Inport  Port=92
  PrkgClimaActvMgrVFCGlobalShortDuration_PrkgClimaActvMgrVFCGlobalShortDuration_read : Inport  Port=93
  PrkgClimaActvMgrVFCLocking_PrkgClimaActvMgrVFCLocking_read : Inport  Port=94
  PrkgClimaActvMgrVFCParkingDrivingClimatization_PrkgClimaActvMgrVFCParkingDrivingClimatization_read : Inport  Port=95
  PrkgClimaActvMgrVFCPostClimatisation_PrkgClimaActvMgrVFCPostClimatisation_read : Inport  Port=96
  PrkgClimaActvMgrVFCWindows_PrkgClimaActvMgrVFCWindows_read : Inport  Port=97
  RlyCmdCmft_RlyCmdCmft_read : Inport  Port=98
  SysServCarCfgSts_SysServCCPBGMNum_read : Inport  Port=99
  VehBattU_VehBattU_read : Inport  Port=100
  VehBattU_VehBattU_IsUpdated_read : Inport  Port=101
  VehModMngtGlbSafe1_Ngcc_VehModMngtGlbSafe1_read : Inport  Port=102
  VFCInfoEna_VFCInfoEna_read : Inport  Port=103
  VFCInfoEna_VFCInfoEna_IsUpdated_read : Inport  Port=104
  function : TriggerPort  TriggerType=function-call  VariantControl=(inherit)  SampleTimeType=periodic  SampleTime=-1
  Ground_{1..5} (5 alike) : Ground
  PA_NvMBlockRecordSignalInit : Constant  Value=NvMBlockRecordSignalInit
  Terminator_{1..105} (105 alike) : Terminator
  CcmIntelliClimaResd1_CcmIntelliClimaResd1_write : Outport
      settings: EnsureOutportIsVirtual=on
  CcmIntelliClimaResd2_CcmIntelliClimaResd2_write : Outport  Port=2
      settings: EnsureOutportIsVirtual=on
  CcmIntelliClimaResd3_CcmIntelliClimaResd3_write : Outport  Port=3
      settings: EnsureOutportIsVirtual=on
  CcmIntelliClimaResd4_CcmIntelliClimaResd4_write : Outport  Port=4
      settings: EnsureOutportIsVirtual=on
  HvacRecFlapActlPosn_HvacRecFlapActlPosn_write : Outport  Port=5
      settings: EnsureOutportIsVirtual=on
  wires:
  ActrPosnFrntActForOsaAndRecirc_ActrPosnFrntActForOsaAndRecirc_read/1 -> Terminator_1/1
  ActrPosnFrntActForRowFirstModDistbn_ActrPosnFrntActForRowFirstModDistbn_read/1 -> Terminator_2/1
  ActrPosnFrntActForRowSecModDistbnLe_ActrPosnFrntActForRowSecModDistbnLe_read/1 -> Terminator_3/1
  ActrPosnFrntActForRowSecModDistbnRi_ActrPosnFrntActForRowSecModDistbnRi_read/1 -> Terminator_4/1
  AirFlapPosnFrntActForRowFirstForActrDefrst_AirFlapPosnFrntActForRowFirstForActrDefrst_read/1 -> Terminator_5/1
  AirFlapPosnFrntActForRowFirstForActrFlrLe_AirFlapPosnFrntActForRowFirstForActrFlrLe_read/1 -> Terminator_6/1
  AirFlapPosnFrntActForRowFirstForActrFlrRi_AirFlapPosnFrntActForRowFirstForActrFlrRi_read/1 -> Terminator_7/1
  AirFlapPosnFrntActForRowFirstForActrTLe_AirFlapPosnFrntActForRowFirstForActrTLe_read/1 -> Terminator_8/1
  AirFlapPosnFrntActForRowFirstForActrTRi_AirFlapPosnFrntActForRowFirstForActrTRi_read/1 -> Terminator_9/1
  AirFlapPosnFrntActForRowFirstForActrVentLe_AirFlapPosnFrntActForRowFirstForActrVentLe_read/1 -> Terminator_10/1
  AirFlapPosnFrntActForRowFirstForActrVentRi_AirFlapPosnFrntActForRowFirstForActrVentRi_read/1 -> Terminator_11/1
  AirFlapPosnFrntActForRowFirstForActrVentSideLe_AirFlapPosnFrntActForRowFirstForActrVentSideLe_read/1 -> Terminator_12/1
  AirFlapPosnFrntActForRowFirstForActrVentSideRi_AirFlapPosnFrntActForRowFirstForActrVentSideRi_read/1 -> Terminator_13/1
  AirFlapPosnFrntActForRowFirstForOsa_AirFlapPosnFrntActForRowFirstForOsa_read/1 -> Terminator_14/1
  AirFlapPosnFrntActForRowFirstForRecirc_AirFlapPosnFrntActForRowFirstForRecirc_read/1 -> Terminator_15/1
  AirFlapPosnFrntActForRowSecForActrTLe_AirFlapPosnFrntActForRowSecForActrTLe_read/1 -> Terminator_16/1
  AirFlapPosnFrntActForRowSecForActrTRi_AirFlapPosnFrntActForRowSecForActrTRi_read/1 -> Terminator_17/1
  BatteryVolValue_BatteryVolValue_read/1 -> Terminator_18/1
  PrkgClimaActvMgrVFCBodyPreClimatisation_PrkgClimaActvMgrVFCBodyPreClimatisation_read/1 -> Terminator_19/1
  FrntHvacBlowerSts_FrntHvacBlowerSts_read/1 -> Terminator_20/1
  HvacOsaAndRecActrQfForHp_HvacOsaAndRecActrQfForHp_read/1 -> Terminator_21/1
  PwrGernalOn2OffCnt_PwrMdGernalOn2OffCnt_read/1 -> Terminator_22/1
  RlyCmftForClimaReq_Ngcc_RlyCmftForClimaReq_read/1 -> Terminator_23/1
  RlyCrashForClimaReq_Ngcc_RlyCrashForClimaReq_read/1 -> Terminator_24/1
  SysServCarCfgSts_SysServCarID_read/1 -> Terminator_25/1
  SysServCarCfgSts_SysServCCPAQSSts_read/1 -> Terminator_26/1
  SysServCarCfgSts_SysServCCPHEXSensorSts_read/1 -> Terminator_27/1
  SysServCarCfgSts_SysServCCPLin2AFUSts_read/1 -> Terminator_28/1
  SysServCarCfgSts_SysServCCPLin2AIUSts_read/1 -> Terminator_29/1
  SysServCarCfgSts_SysServCCPLin2CDSSts_read/1 -> Terminator_30/1
  SysServCarCfgSts_SysServCCPLin2PMSISts_read/1 -> Terminator_31/1
  SysServCarCfgSts_SysServCCPLin3VGASts_read/1 -> Terminator_32/1
  SysServCarCfgSts_SysServDUAirVentSnrSts_read/1 -> Terminator_33/1
  SysServCarCfgSts_SysServDUBlowerPwmSignSts_read/1 -> Terminator_34/1
  SysServCarCfgSts_SysServDUBlowerPwmSts_read/1 -> Terminator_35/1
  SysServCarCfgSts_SysServDUDCDefrostSts_read/1 -> Terminator_36/1
  SysServCarCfgSts_SysServDUDCFLMdeSts_read/1 -> Terminator_37/1
  SysServCarCfgSts_SysServDUDCModeSts_read/1 -> Terminator_38/1
  SysServCarCfgSts_SysServDUDCRecSts_read/1 -> Terminator_39/1
  SysServCarCfgSts_SysServDUDCSModeSts_read/1 -> Terminator_40/1
  SysServCarCfgSts_SysServDUDCSTLSts_read/1 -> Terminator_41/1
  SysServCarCfgSts_SysServDUDCTLSts_read/1 -> Terminator_42/1
  SysServCarCfgSts_SysServDUDCTRSts_read/1 -> Terminator_43/1
  SysServCarCfgSts_SysServDUFLFootSts_read/1 -> Terminator_44/1
  SysServCarCfgSts_SysServDUFLVentSts_read/1 -> Terminator_45/1
  SysServCarCfgSts_SysServDUFRFootSts_read/1 -> Terminator_46/1
  SysServCarCfgSts_SysServDUFRVentSts_read/1 -> Terminator_47/1
  SysServCarCfgSts_SysServDUIncarMotorSts_read/1 -> Terminator_48/1
  SysServCarCfgSts_SysServDULin1HBMFSts_read/1 -> Terminator_49/1
  SysServCarCfgSts_SysServDULin4HFAFSts_read/1 -> Terminator_50/1
  SysServCarCfgSts_SysServDUSFLFootSts_read/1 -> Terminator_51/1
  SysServCarCfgSts_SysServDUSFLVentSts_read/1 -> Terminator_52/1
  SysServCarCfgSts_SysServDUSFRFootSts_read/1 -> Terminator_53/1
  SysServCarCfgSts_SysServDUSFRVentSts_read/1 -> Terminator_54/1
  SysServCarCfgSts_SysServDUSnsrForFCmptmtTSts_read/1 -> Terminator_55/1
  SysServCarCfgSts_SysServDUSnsrForRair_read/1 -> Terminator_56/1
  SysServCarCfgSts_SysServDUSnsrForRCmptmtTSts_read/1 -> Terminator_57/1
  VehSpdLgt_Ngcc_VehSpdLgt_read/1 -> Terminator_58/1
  CmptmtTFrntRaw_CmptmtTFrntRaw_read/1 -> Terminator_59/1
  AirFragCh2RunngSts_AirFragCh2RunngSts_read/1 -> Terminator_60/1
  AirFragCh2RunngSts_AirFragCh2RunngSts_IsUpdated_read/1 -> Terminator_61/1
  AirFragCh3RunngSts_AirFragCh3RunngSts_read/1 -> Terminator_62/1
  AirFragCh3RunngSts_AirFragCh3RunngSts_IsUpdated_read/1 -> Terminator_63/1
  AirFragCh4RunngSts_AirFragCh4RunngSts_read/1 -> Terminator_64/1
  AirFragCh4RunngSts_AirFragCh4RunngSts_IsUpdated_read/1 -> Terminator_65/1
  AirFragCh5RunngSts_AirFragCh5RunngSts_read/1 -> Terminator_66/1
  AirFragCh5RunngSts_AirFragCh5RunngSts_IsUpdated_read/1 -> Terminator_67/1
  HvacFanSts2HvacFanBattI2_HvacFanSts2HvacFanBattI2_read/1 -> Terminator_68/1
  HvacFanSts2HvacFanBattI2_HvacFanSts2HvacFanBattI2_IsUpdated_read/1 -> Terminator_69/1
  HvacFanSts2HvacFanBattU2_HvacFanSts2HvacFanBattU2_read/1 -> Terminator_70/1
  HvacFanSts2HvacFanBattU2_HvacFanSts2HvacFanBattU2_IsUpdated_read/1 -> Terminator_71/1
  HvacFanSts3HvacFanBattI_HvacFanSts3HvacFanBattI_read/1 -> Terminator_72/1
  HvacFanSts3HvacFanBattI_HvacFanSts3HvacFanBattI_IsUpdated_read/1 -> Terminator_73/1
  HvacFanSts3HvacFanBattU_HvacFanSts3HvacFanBattU_read/1 -> Terminator_74/1
  HvacFanSts3HvacFanBattU_HvacFanSts3HvacFanBattU_IsUpdated_read/1 -> Terminator_75/1
  HvacFanSts3HvacFanSpdFd_HvacFanSts3HvacFanSpdFd_read/1 -> Terminator_76/1
  HvacFanSts3HvacFanSpdFd_HvacFanSts3HvacFanSpdFd_IsUpdated_read/1 -> Terminator_77/1
  IntCo2Conc_IntCo2Conc_read/1 -> Terminator_78/1
  IntCo2Conc_IntCo2Conc_IsUpdated_read/1 -> Terminator_79/1
  IntCo2RunSts_IntCo2RunSts_read/1 -> Terminator_80/1
  IntCo2RunSts_IntCo2RunSts_IsUpdated_read/1 -> Terminator_81/1
  AirFragCh1RunngSts_AirFragCh1RunngSts_read/1 -> Terminator_82/1
  AirFragCh1RunngSts_AirFragCh1RunngSts_IsUpdated_read/1 -> Terminator_83/1
  AmbTRaw_AmbTRaw_read/1 -> Terminator_84/1
  BkpOfDstTrvld_BkpOfDstTrvld_read/1 -> Terminator_85/1
  BkpOfDstTrvld_BkpOfDstTrvld_IsUpdated_read/1 -> Terminator_86/1
  CarTiGlb_CarTiGlb_read/1 -> Terminator_87/1
  CarTiGlb_CarTiGlb_IsUpdated_read/1 -> Terminator_88/1
  ClimaTiMgrVFCInfotainmentPush_ClimaTiMgrVFCInfotainmentPush_read/1 -> Terminator_89/1
  ClimRlyCmd_ClimRlyCmd_read/1 -> Terminator_90/1
  CmptmtTRearRaw_CmptmtTRearRaw_read/1 -> Terminator_91/1
  HmiHvacFanLvlFrnt_HmiHvacFanLvlFrnt_read/1 -> Terminator_92/1
  PrkgClimaActvMgrVFCGlobalShortDuration_PrkgClimaActvMgrVFCGlobalShortDuration_read/1 -> Terminator_93/1
  PrkgClimaActvMgrVFCLocking_PrkgClimaActvMgrVFCLocking_read/1 -> Terminator_94/1
  PrkgClimaActvMgrVFCParkingDrivingClimatization_PrkgClimaActvMgrVFCParkingDrivingClimatization_read/1 -> Terminator_95/1
  PrkgClimaActvMgrVFCPostClimatisation_PrkgClimaActvMgrVFCPostClimatisation_read/1 -> Terminator_96/1
  PrkgClimaActvMgrVFCWindows_PrkgClimaActvMgrVFCWindows_read/1 -> Terminator_97/1
  RlyCmdCmft_RlyCmdCmft_read/1 -> Terminator_98/1
  SysServCarCfgSts_SysServCCPBGMNum_read/1 -> Terminator_99/1
  VehBattU_VehBattU_read/1 -> Terminator_100/1
  VehBattU_VehBattU_IsUpdated_read/1 -> Terminator_101/1
  VehModMngtGlbSafe1_Ngcc_VehModMngtGlbSafe1_read/1 -> Terminator_102/1
  VFCInfoEna_VFCInfoEna_read/1 -> Terminator_103/1
  VFCInfoEna_VFCInfoEna_IsUpdated_read/1 -> Terminator_104/1
  PA_NvMBlockRecordSignalInit/1 -> Terminator_105/1
  Ground_1/1 -> CcmIntelliClimaResd1_CcmIntelliClimaResd1_write/1
  Ground_2/1 -> CcmIntelliClimaResd2_CcmIntelliClimaResd2_write/1
  Ground_3/1 -> CcmIntelliClimaResd3_CcmIntelliClimaResd3_write/1
  Ground_4/1 -> CcmIntelliClimaResd4_CcmIntelliClimaResd4_write/1
  Ground_5/1 -> HvacRecFlapActlPosn_HvacRecFlapActlPosn_write/1

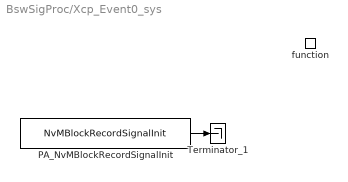
== system BswSigProc/Xcp_Event0_sys ==
  function : TriggerPort  TriggerType=function-call  VariantControl=(inherit)  SampleTimeType=periodic  SampleTime=-1
  PA_NvMBlockRecordSignalInit : Constant  Value=NvMBlockRecordSignalInit
  Terminator_1 : Terminator
  wires:
  PA_NvMBlockRecordSignalInit/1 -> Terminator_1/1

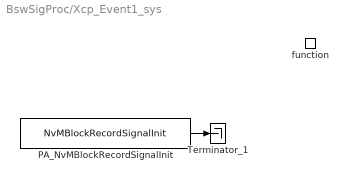
== system BswSigProc/Xcp_Event1_sys ==
  function : TriggerPort  TriggerType=function-call  VariantControl=(inherit)  SampleTimeType=periodic  SampleTime=-1
  PA_NvMBlockRecordSignalInit : Constant  Value=NvMBlockRecordSignalInit
  Terminator_1 : Terminator
  wires:
  PA_NvMBlockRecordSignalInit/1 -> Terminator_1/1

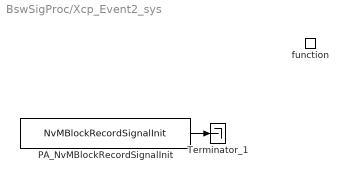
== system BswSigProc/Xcp_Event2_sys ==
  function : TriggerPort  TriggerType=function-call  VariantControl=(inherit)  SampleTimeType=periodic  SampleTime=-1
  PA_NvMBlockRecordSignalInit : Constant  Value=NvMBlockRecordSignalInit
  Terminator_1 : Terminator
  wires:
  PA_NvMBlockRecordSignalInit/1 -> Terminator_1/1

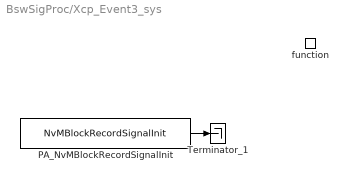
== system BswSigProc/Xcp_Event3_sys ==
  function : TriggerPort  TriggerType=function-call  VariantControl=(inherit)  SampleTimeType=periodic  SampleTime=-1
  PA_NvMBlockRecordSignalInit : Constant  Value=NvMBlockRecordSignalInit
  Terminator_1 : Terminator
  wires:
  PA_NvMBlockRecordSignalInit/1 -> Terminator_1/1

== system BswSigProc/Xcp_Event4_sys ==
  function : TriggerPort  TriggerType=function-call  VariantControl=(inherit)  SampleTimeType=periodic  SampleTime=-1
  PA_NvMBlockRecordSignalInit : Constant  Value=NvMBlockRecordSignalInit
  Terminator_1 : Terminator
  wires:
  PA_NvMBlockRecordSignalInit/1 -> Terminator_1/1

== system BswSigProc/Xcp_Event5_sys ==
  function : TriggerPort  TriggerType=function-call  VariantControl=(inherit)  SampleTimeType=periodic  SampleTime=-1
  PA_NvMBlockRecordSignalInit : Constant  Value=NvMBlockRecordSignalInit
  Terminator_1 : Terminator
  wires:
  PA_NvMBlockRecordSignalInit/1 -> Terminator_1/1

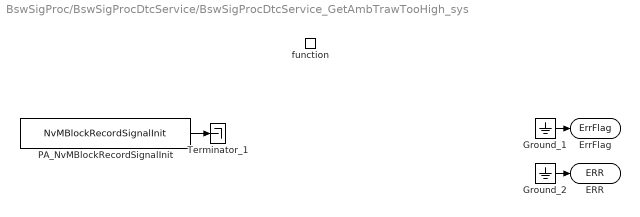
== system BswSigProc/BswSigProcDtcService/BswSigProcDtcService_GetAmbTrawTooHigh_sys ==
  function : TriggerPort  TriggerType=function-call  IsSimulinkFunction=on  FunctionName=BswSigProcDtcService_GetAmbTrawTooHigh  VariantControl=(inherit)
  Ground_1 : Ground
  Ground_2 : Ground
  PA_NvMBlockRecordSignalInit : Constant  Value=NvMBlockRecordSignalInit
  Terminator_1 : Terminator
  ErrFlag : ArgOut  OutDataTypeStr=Enum: DTCState  ArgumentName=ErrFlag
  ERR : ArgOut  Port=2  OutDataTypeStr=Std_ReturnType  ArgumentName=ERR
  wires:
  PA_NvMBlockRecordSignalInit/1 -> Terminator_1/1
  Ground_1/1 -> ErrFlag/1
  Ground_2/1 -> ERR/1

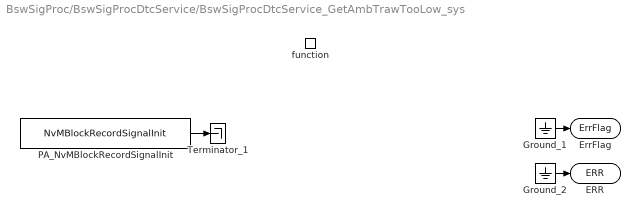
== system BswSigProc/BswSigProcDtcService/BswSigProcDtcService_GetAmbTrawTooLow_sys ==
  function : TriggerPort  TriggerType=function-call  IsSimulinkFunction=on  FunctionName=BswSigProcDtcService_GetAmbTrawTooLow  VariantControl=(inherit)
  Ground_1 : Ground
  Ground_2 : Ground
  PA_NvMBlockRecordSignalInit : Constant  Value=NvMBlockRecordSignalInit
  Terminator_1 : Terminator
  ErrFlag : ArgOut  OutDataTypeStr=Enum: DTCState  ArgumentName=ErrFlag
  ERR : ArgOut  Port=2  OutDataTypeStr=Std_ReturnType  ArgumentName=ERR
  wires:
  PA_NvMBlockRecordSignalInit/1 -> Terminator_1/1
  Ground_1/1 -> ErrFlag/1
  Ground_2/1 -> ERR/1

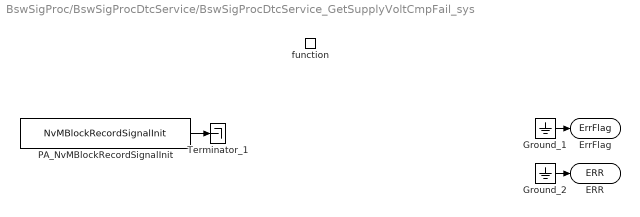
== system BswSigProc/BswSigProcDtcService/BswSigProcDtcService_GetSupplyVoltCmpFail_sys ==
  function : TriggerPort  TriggerType=function-call  IsSimulinkFunction=on  FunctionName=BswSigProcDtcService_GetSupplyVoltCmpFail  VariantControl=(inherit)
  Ground_1 : Ground
  Ground_2 : Ground
  PA_NvMBlockRecordSignalInit : Constant  Value=NvMBlockRecordSignalInit
  Terminator_1 : Terminator
  ErrFlag : ArgOut  OutDataTypeStr=Enum: DTCState  ArgumentName=ErrFlag
  ERR : ArgOut  Port=2  OutDataTypeStr=Std_ReturnType  ArgumentName=ERR
  wires:
  PA_NvMBlockRecordSignalInit/1 -> Terminator_1/1
  Ground_1/1 -> ErrFlag/1
  Ground_2/1 -> ERR/1

== system BswSigProc/BswSigProc_Runnable_sys/Ground_12 ==
  Ground_12 : Ground
  Out1 : Outport  OutDataTypeStr=Bus: CmptmtTFrnt  PortDimensions=1
  wires:
  Ground_12/1 -> Out1/1

== system BswSigProc/BswSigProc_Runnable_sys/Ground_13 ==
  Ground_13 : Ground
  Out1 : Outport  OutDataTypeStr=Bus: EvaprTFrnt  PortDimensions=1
  wires:
  Ground_13/1 -> Out1/1

== system BswSigProc/BswSigProc_Runnable_sys/Ground_15 ==
  Ground_15 : Ground
  Out1 : Outport  OutDataTypeStr=Bus: HvacAirTForHeatrFrnt  PortDimensions=1
  wires:
  Ground_15/1 -> Out1/1

== system BswSigProc/BswSigProc_Runnable_sys/Ground_16 ==
  Ground_16 : Ground
  Out1 : Outport  OutDataTypeStr=Bus: VFCVector  PortDimensions=1
  wires:
  Ground_16/1 -> Out1/1

== system BswSigProc/BswSigProc_Runnable_sys/Ground_21 ==
  Ground_21 : Ground
  Out1 : Outport  OutDataTypeStr=Bus: CmptmtTFrnt  PortDimensions=1
  wires:
  Ground_21/1 -> Out1/1

== system BswSigProc/BswSigProc_Runnable_sys/Ground_22 ==
  Ground_22 : Ground
  Out1 : Outport  OutDataTypeStr=Bus: PNCState  PortDimensions=1
  wires:
  Ground_22/1 -> Out1/1

== system BswSigProc/BswSigProc_Runnable_sys/Ground_25 ==
  Ground_25 : Ground
  Out1 : Outport  OutDataTypeStr=Bus: EvaprTFrnt  PortDimensions=1
  wires:
  Ground_25/1 -> Out1/1
